simulink model "TannenwegFinal"  (R2024a)
settings: Solver=ode15s  StartTime=0.0  StopTime=3600*3  FixedStep=0.1  RelTol=0.001
model workspace (MAT data in the model file):
  Ti = 12
  ips = 36
  roomarea1 = 20
  s = 30
libraries: fl_lib x1521, nesl_utility x126, SimscapeFluids_lib x35
(part 24 of 24)

== system TannenwegFinal/OG2/Raum5/Heater/Subsystem ==
  VCmd : Inport
  Gain : Gain  Gain=0.1
  Selector : Selector  InputPortWidth=36  IndexOptions=Index vector (dialog)  Indices=29  OutputSizes=1
  Simulink-PS Converter : nesl_utility/Simulink-PS Converter  PhysicalDomain=network_engine_domain  SubClassName=ps_input  LeftPortType=input  RightPortType=output  PseudoPeriodic=off  Frequencies=[]  NoiseDistribution=none  NoiseParameters=[]  Unit=1  AffineConversion=off  FilteringAndDerivatives=provide  SimscapeFilterOrder=1  InputFilterTimeConstant=0.001  UdotUserProvided=0
  Transfer Fcn : TransferFcn  Denominator=[s 1]
  output : PMIOPort  Side=Right
  wires:
  Transfer Fcn/1 -> Simulink-PS Converter/1
  Gain/1 -> Transfer Fcn/1
  Selector/1 -> Gain/1
  VCmd/1 -> Selector/1
  connections:
  Simulink-PS Converter/RConn1 -- output/RConn1

== system TannenwegFinal/OG2/Raum6/Heater/Subsystem ==
  VCmd : Inport
  Gain : Gain  Gain=0.1
  Selector : Selector  InputPortWidth=36  IndexOptions=Index vector (dialog)  Indices=30  OutputSizes=1
  Simulink-PS Converter : nesl_utility/Simulink-PS Converter  PhysicalDomain=network_engine_domain  SubClassName=ps_input  LeftPortType=input  RightPortType=output  PseudoPeriodic=off  Frequencies=[]  NoiseDistribution=none  NoiseParameters=[]  Unit=1  AffineConversion=off  FilteringAndDerivatives=provide  SimscapeFilterOrder=1  InputFilterTimeConstant=0.001  UdotUserProvided=0
  Transfer Fcn : TransferFcn  Denominator=[s 1]
  output : PMIOPort  Side=Right
  wires:
  Transfer Fcn/1 -> Simulink-PS Converter/1
  Gain/1 -> Transfer Fcn/1
  Selector/1 -> Gain/1
  VCmd/1 -> Selector/1
  connections:
  Simulink-PS Converter/RConn1 -- output/RConn1

== system TannenwegFinal/OG2/Raum7/Heater/Subsystem ==
  VCmd : Inport
  Gain : Gain  Gain=0.1
  Scope : Scope  NumInputPorts=2
  Selector : Selector  InputPortWidth=36  IndexOptions=Index vector (dialog)  Indices=31  OutputSizes=1
  Simulink-PS Converter : nesl_utility/Simulink-PS Converter  PhysicalDomain=network_engine_domain  SubClassName=ps_input  LeftPortType=input  RightPortType=output  PseudoPeriodic=off  Frequencies=[]  NoiseDistribution=none  NoiseParameters=[]  Unit=1  AffineConversion=off  FilteringAndDerivatives=provide  SimscapeFilterOrder=1  InputFilterTimeConstant=0.001  UdotUserProvided=0
  Transfer Fcn : TransferFcn  Denominator=[s 1]
  output : PMIOPort  Side=Right
  wires:
  Transfer Fcn/1 -> Simulink-PS Converter/1, Scope/1
  Gain/1 -> Scope/2, Transfer Fcn/1
  Selector/1 -> Gain/1
  VCmd/1 -> Selector/1
  connections:
  Simulink-PS Converter/RConn1 -- output/RConn1

== system TannenwegFinal/OG2/Raum8/Heater/Subsystem ==
  VCmd : Inport
  Gain : Gain  Gain=0.1
  Selector : Selector  InputPortWidth=36  IndexOptions=Index vector (dialog)  Indices=32  OutputSizes=1
  Simulink-PS Converter : nesl_utility/Simulink-PS Converter  PhysicalDomain=network_engine_domain  SubClassName=ps_input  LeftPortType=input  RightPortType=output  PseudoPeriodic=off  Frequencies=[]  NoiseDistribution=none  NoiseParameters=[]  Unit=1  AffineConversion=off  FilteringAndDerivatives=provide  SimscapeFilterOrder=1  InputFilterTimeConstant=0.001  UdotUserProvided=0
  Transfer Fcn : TransferFcn  Denominator=[s 1]
  output : PMIOPort  Side=Right
  wires:
  Transfer Fcn/1 -> Simulink-PS Converter/1
  Gain/1 -> Transfer Fcn/1
  Selector/1 -> Gain/1
  VCmd/1 -> Selector/1
  connections:
  Simulink-PS Converter/RConn1 -- output/RConn1

== system TannenwegFinal/OG2/Raum9/Heater/Subsystem ==
  VCmd : Inport
  Gain : Gain  Gain=0.1
  Selector : Selector  InputPortWidth=36  IndexOptions=Index vector (dialog)  Indices=33  OutputSizes=1
  Simulink-PS Converter : nesl_utility/Simulink-PS Converter  PhysicalDomain=network_engine_domain  SubClassName=ps_input  LeftPortType=input  RightPortType=output  PseudoPeriodic=off  Frequencies=[]  NoiseDistribution=none  NoiseParameters=[]  Unit=1  AffineConversion=off  FilteringAndDerivatives=provide  SimscapeFilterOrder=1  InputFilterTimeConstant=0.001  UdotUserProvided=0
  Transfer Fcn : TransferFcn  Denominator=[s 1]
  output : PMIOPort  Side=Right
  wires:
  Transfer Fcn/1 -> Simulink-PS Converter/1
  Gain/1 -> Transfer Fcn/1
  Selector/1 -> Gain/1
  VCmd/1 -> Selector/1
  connections:
  Simulink-PS Converter/RConn1 -- output/RConn1

== matlab function TannenwegFinal/MATLAB Function ==
  data: TroomArray input; valveStates output; TdesiredArray input; Taverage output
function [valveStates, Taverage] = controlAllValves(TroomArray, TdesiredArray)
% controlAllValves computes valve commands and Taverage for multiple rooms.
%
% Inputs:
%   TroomArray     - Nx1 or 1xN vector of current room temperatures
%   TdesiredArray  - Nx1 or 1xN vector of desired temperatures
%
% Outputs:
%   valveStates    - Nx1 double array (1 = open, 0 = closed)
%   Taverage       - Scalar double, average temp of rooms needing heating

    % Ensure column vectors
    TroomArray = TroomArray(:);
    TdesiredArray = TdesiredArray(:);

    % Number of rooms
    nRooms = length(TroomArray);

    % Initialize valve states
    valveStates = zeros(nRooms, 1);  % Default to closed

    % Compute dynamic Taverage
    heatingNeededIdx = TroomArray+0.3< TdesiredArray;

    if any(heatingNeededIdx)
        Taverage = mean(TroomArray(heatingNeededIdx));
    else
        Taverage = NaN;  % Or fallback value
    end

    % Apply control logic
    for i = 1:nRooms
        Troom = TroomArray(i);
        Tdesired = TdesiredArray(i);

        if Troom < Tdesired
            if Troom-0.1< Taverage
                valveStates(i) = 1;  % open
            else
                valveStates(i) = 0;  % closed
            end
        else
            valveStates(i) = 0;  % closed
        end
    end
end
